simulink library "lib_all"  (Simulink 7.6)
settings: Solver=ode45  SolverMode=SingleTasking  StartTime=0.0  StopTime=10.0  RelTol=1e-3
values from neighbouring files (symbols this model uses):
  map_lib_03 = [6.8679 7.0159 7.4571 8.1849 9.1892 10.456 11.97 13.71 15.655 17.781 20.063 22.474 ... (803 elements, 73x11)]    [scr_simdata.mat]    (also in scr_simdata_all.mat)
  map_lib_06 = [2.5784 2.6727 2.9588 3.4453 4.1444 5.068 6.226 7.6242 9.2634 11.139 13.24 15.552 ... (803 elements, 73x11)]    [scr_simdata.mat]    (also in scr_simdata_all.mat)
  map_lib_09 = [0.0025784 0.0026727 0.0029588 0.0034453 0.0041444 0.005068 0.006226 0.0076242 0.0092634 0.011139 0.01324 0.015552 ... (803 elements, 73x11)]    [scr_simdata.mat]    (also in scr_simdata_all.mat)
  map_lib_10 = [2.74 2.75 2.76 2.77 2.78 2.79 2.8 2.81 2.82 2.83 2.84 2.85 ... (81 elements, 1x81)]    [scr_simdata.mat]    (also in scr_simdata_all.mat)
  map_lib_11 = [1.1983 1.2027 1.207 1.2113 1.2156 1.2198 1.2241 1.2283 1.2326 1.2368 1.241 1.2451 ... (81 elements, 1x81)]    [scr_simdata.mat]    (also in scr_simdata_all.mat)
  map_lib_12 = [2.74 2.75 2.76 2.77 2.78 2.79 2.8 2.81 2.82 2.83 2.84 2.85 ... (81 elements, 1x81)]    [scr_simdata.mat]    (also in scr_simdata_all.mat)
  map_lib_13 = [1.74 1.75 1.76 1.77 1.78 1.79 1.8 1.81 1.82 1.83 1.84 1.85 ... (81 elements, 1x81)]    [scr_simdata.mat]    (also in scr_simdata_all.mat)
  map_lib_16 = [0.00048828125 1.6159 1.8962 2.0821 2.2251 2.3426 2.4433 2.5318 2.611 2.683 2.749 2.8101 ... (1419 elements, 129x11)]    [scr_simdata.mat]    (also in scr_simdata_all.mat)
  map_mr2_01 = [0 800 1600 2400 3200 4000]    [scr_simdata.mat]    (also in scr_simdata_all.mat)
  map_mr2_02 = [1.32 1.32 1.33 1.34 1.36 1.38]    [scr_simdata.mat]    (also in scr_simdata_all.mat)
  map_mr2_03 = [0 800 1600 2400 3200 4000]    [scr_simdata.mat]    (also in scr_simdata_all.mat)
  map_mr2_04 = [1.32 1.32 1.33 1.34 1.36 1.38]    [scr_simdata.mat]    (also in scr_simdata_all.mat)
  map_mr2_05 = [0 800 1600 2400 3200 4000]    [scr_simdata.mat]    (also in scr_simdata_all.mat)
  map_mr2_06 = [1.32 1.32 1.33 1.34 1.36 1.38]    [scr_simdata.mat]    (also in scr_simdata_all.mat)
  Parameter1 = 6    [scr_simdata.mat]    (also in scr_simdata_all.mat)
  Parameter101 = 30    [scr_simdata.mat]    (also in scr_simdata_all.mat)
  Parameter102 = 12    [scr_simdata.mat]    (also in scr_simdata_all.mat)
  Parameter103 = -240    [scr_simdata.mat]    (also in scr_simdata_all.mat)
  Parameter108 = 48    [scr_simdata.mat]    (also in scr_simdata_all.mat)
  Parameter120 = 16    [scr_simdata.mat]    (also in scr_simdata_all.mat)
  Parameter121 = 87    [scr_simdata.mat]    (also in scr_simdata_all.mat)
  Parameter123 = 78    [scr_simdata.mat]    (also in scr_simdata_all.mat)
  Parameter124 = 60    [scr_simdata.mat]    (also in scr_simdata_all.mat)
  Parameter125 = -480    [scr_simdata.mat]    (also in scr_simdata_all.mat)
  Parameter130 = 96    [scr_simdata.mat]    (also in scr_simdata_all.mat)
  Parameter131 = 3    [scr_simdata.mat]    (also in scr_simdata_all.mat)
  Parameter144 = 3    [scr_simdata.mat]    (also in scr_simdata_all.mat)
  Parameter18 = 128    [scr_simdata.mat]    (also in scr_simdata_all.mat)
  Parameter19 = 16    [scr_simdata.mat]    (also in scr_simdata_all.mat)
  Parameter2 = 12    [scr_simdata.mat]    (also in scr_simdata_all.mat)
  Parameter20 = 7    [scr_simdata.mat]    (also in scr_simdata_all.mat)
  Parameter21 = 4    [scr_simdata.mat]    (also in scr_simdata_all.mat)
  Parameter3 = 18    [scr_simdata.mat]    (also in scr_simdata_all.mat)
  Parameter32 = 1    [scr_simdata.mat]    (also in scr_simdata_all.mat)
  Parameter34 = 24    [scr_simdata.mat]    (also in scr_simdata_all.mat)
  Parameter4 = 138    [scr_simdata.mat]    (also in scr_simdata_all.mat)
  Parameter42 = 48    [scr_simdata.mat]    (also in scr_simdata_all.mat)
  Parameter5 = 135    [scr_simdata.mat]    (also in scr_simdata_all.mat)
  Parameter55 = 0    [scr_simdata.mat]    (also in scr_simdata_all.mat)
  Parameter56 = 0    [scr_simdata.mat]    (also in scr_simdata_all.mat)
  Parameter57 = 1    [scr_simdata.mat]    (also in scr_simdata_all.mat)
  Parameter58 = 1    [scr_simdata.mat]    (also in scr_simdata_all.mat)
  Parameter59 = 2    [scr_simdata.mat]    (also in scr_simdata_all.mat)
  Parameter6 = 132    [scr_simdata.mat]    (also in scr_simdata_all.mat)
  Parameter60 = 2    [scr_simdata.mat]    (also in scr_simdata_all.mat)
  Parameter63 = 144    [scr_simdata.mat]    (also in scr_simdata_all.mat)
  Parameter7 = 126    [scr_simdata.mat]    (also in scr_simdata_all.mat)
  Parameter76 = 64    [scr_simdata.mat]    (also in scr_simdata_all.mat)
  Parameter77 = 135    [scr_simdata.mat]    (also in scr_simdata_all.mat)
  Parameter79 = 126    [scr_simdata.mat]    (also in scr_simdata_all.mat)
  Parameter80 = 108    [scr_simdata.mat]    (also in scr_simdata_all.mat)
  Parameter81 = 0    [scr_simdata.mat]    (also in scr_simdata_all.mat)
  Parameter86 = 0    [scr_simdata.mat]    (also in scr_simdata_all.mat)
  Parameter98 = 112    [scr_simdata.mat]    (also in scr_simdata_all.mat)
  Parameter99 = 39    [scr_simdata.mat]    (also in scr_simdata_all.mat)
undefined symbols (not defined in the file or in its folder): cylvolkp_hih_map2h, cylvolkp_map2h, pk1k_map2h
libraries: simulink x2
(part 2 of 36)

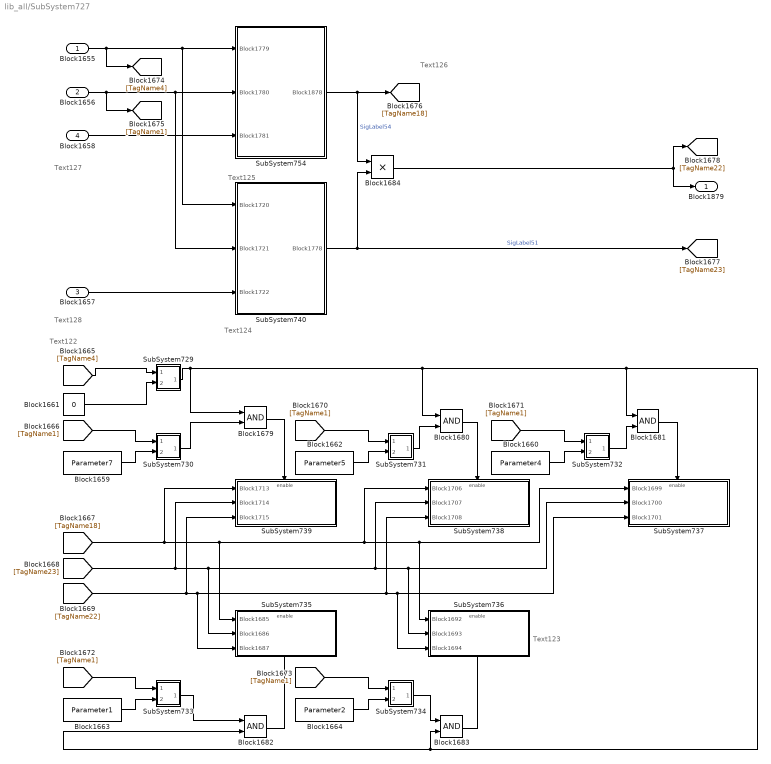
== system lib_all/SubSystem727 ==
  note: Text128
  note: Text127
  note: Text126
  note: Text125
  note: Text124
  note: Text123
  note: Text122
  Block1655 : Inport
  Block1656 : Inport  Port=2
  Block1657 : Inport  Port=3
  Block1658 : Inport  Port=4
  Block1659 : Constant  Value=Parameter7  OutDataTypeStr=double  SampleTime=-1
  Block1660 : Constant  Value=Parameter4  OutDataTypeStr=double  SampleTime=-1
  Block1661 : Constant  Value=0  OutDataTypeStr=double  SampleTime=-1
  Block1662 : Constant  Value=Parameter5  OutDataTypeStr=double  SampleTime=-1
  Block1663 : Constant  Value=Parameter1  OutDataTypeStr=double  SampleTime=-1
  Block1664 : Constant  Value=Parameter2  OutDataTypeStr=double  SampleTime=-1
  Block{1679..1683} (5 alike) : Logic
  Block1684 : Product  OutDataTypeStr=double  SaturateOnIntegerOverflow=on  RndMeth=Zero  InputSameDT=on
  SubSystem729 : Subsystem
  SubSystem730 : Subsystem
  SubSystem731 : Subsystem
  SubSystem732 : Subsystem
  SubSystem733 : Subsystem
  SubSystem734 : Subsystem
  SubSystem735 : Subsystem
      settings: PropExecContextOutsideSubsystem=on
  SubSystem736 : Subsystem
      settings: PropExecContextOutsideSubsystem=on
  SubSystem737 : Subsystem
      settings: PropExecContextOutsideSubsystem=on
  SubSystem738 : Subsystem
      settings: PropExecContextOutsideSubsystem=on
  SubSystem739 : Subsystem
      settings: PropExecContextOutsideSubsystem=on
  SubSystem740 : Subsystem  Priority=2  TreatAsAtomicUnit=on
      settings: AttributesFormatString=Attribute349  RTWFcnNameOpts=Use subsystem name
  SubSystem754 : Subsystem  Priority=1  TreatAsAtomicUnit=on
      settings: AttributesFormatString=Attribute363  RTWFcnNameOpts=Use subsystem name
  Block1879 : Outport
  wires:
  Block1657/1 -> SubSystem740/3(Block1722)
  Block1658/1 -> SubSystem754/3(Block1781)
  Block1655/1 -> [TagName4], SubSystem754/1(Block1779), SubSystem740/1(Block1720)
  Block1656/1 -> [TagName1], SubSystem754/2(Block1780), SubSystem740/2(Block1721)
  SubSystem754/1(Block1878) -> [TagName18], Block1684/1  "SigLabel54"
  SubSystem740/1(Block1778) -> [TagName23], Block1684/2  "SigLabel51"
  Block1684/1 -> [TagName22], Block1879/1
  [TagName4] -> SubSystem729/1(In1)
  Block1661/1 -> SubSystem729/2(In2)
  [TagName1] -> SubSystem730/1(In1)
  Block1659/1 -> SubSystem730/2(In2)
  SubSystem729/1(Out1) -> Block1682/2, Block1683/2, Block1681/1, Block1680/1, Block1679/1
  SubSystem730/1(Out1) -> Block1679/2
  [TagName1] -> SubSystem731/1(In1)
  Block1662/1 -> SubSystem731/2(In2)
  SubSystem731/1(Out1) -> Block1680/2
  Block1679/1 -> SubSystem739/Enable
  [TagName1] -> SubSystem732/1(In1)
  Block1660/1 -> SubSystem732/2(In2)
  SubSystem732/1(Out1) -> Block1681/2
  Block1680/1 -> SubSystem738/Enable
  Block1681/1 -> SubSystem737/Enable
  [TagName18] -> SubSystem738/1(Block1706), SubSystem736/1(Block1692), SubSystem737/1(Block1699), SubSystem735/1(Block1685), SubSystem739/1(Block1713)
  [TagName23] -> SubSystem738/2(Block1707), SubSystem736/2(Block1693), SubSystem737/2(Block1700), SubSystem735/2(Block1686), SubSystem739/2(Block1714)
  [TagName22] -> SubSystem738/3(Block1708), SubSystem736/3(Block1694), SubSystem737/3(Block1701), SubSystem735/3(Block1687), SubSystem739/3(Block1715)
  [TagName1] -> SubSystem733/1(In1)
  Block1663/1 -> SubSystem733/2(In2)
  [TagName1] -> SubSystem734/1(In1)
  Block1664/1 -> SubSystem734/2(In2)
  Block1682/1 -> SubSystem735/Enable
  Block1683/1 -> SubSystem736/Enable
  SubSystem733/1(Out1) -> Block1682/1
  SubSystem734/1(Out1) -> Block1683/1

== system lib_all/SubSystem795 ==
  note: Text129
  Block1880 : Inport
  Block1881 : Inport  Port=2
  Block1882 : Inport  Port=3
  Block{1883,1885,1887..1888} (4 alike) : Constant  OutDataTypeStr=double  SampleTime=-1
  Block1884 : Constant  Value=0  OutDataTypeStr=double  SampleTime=-1
  Block1886 : Constant  Value=0  OutDataTypeStr=double  SampleTime=-1
  Block1894 : Sum  OutDataTypeStr=double  RndMeth=Zero  SaturateOnIntegerOverflow=on  InputSameDT=on
  Block1895 : Sum  Inputs=+++  OutDataTypeStr=double  RndMeth=Zero  SaturateOnIntegerOverflow=on  InputSameDT=on
  Block1896 : Sum  Inputs=+++  OutDataTypeStr=double  RndMeth=Zero  SaturateOnIntegerOverflow=on  InputSameDT=on
  Block1897 : Sum  Inputs=--+  OutDataTypeStr=double  RndMeth=Zero  SaturateOnIntegerOverflow=on  InputSameDT=on
  Block1898 : Sum  Inputs=--+  OutDataTypeStr=double  RndMeth=Zero  SaturateOnIntegerOverflow=on  InputSameDT=on
  Block{1899..1902} (4 alike) : Switch  Threshold=0.5  RndMeth=Zero  SaturateOnIntegerOverflow=on  InputSameDT=on
  SubSystem796 : Subsystem
  SubSystem797 : Subsystem
  SubSystem798 : Subsystem
  SubSystem799 : Subsystem
  Block1903 : Outport
  wires:
  Block1880/1 -> [TagName14]
  SubSystem796/1(Out1) -> Block1900/2
  Block1884/1 -> SubSystem796/1(In1)
  Block1895/1 -> Block1896/3, Block1899/3, SubSystem797/2(In2)
  Block1883/1 -> Block1895/2
  [TagName14] -> Block1895/1, Block1896/1
  SubSystem797/1(Out1) -> Block1899/2
  Block1886/1 -> SubSystem797/1(In1)
  Block1896/1 -> Block1899/1
  Block1885/1 -> Block1896/2
  Block1899/1 -> Block1900/1
  SubSystem798/1(Out1) -> Block1901/2
  [TagName14] -> SubSystem798/1(In1)
  Block1897/1 -> Block1901/1
  Block1887/1 -> Block1897/2
  [TagName14] -> Block1897/1, Block1898/1
  SubSystem799/1(Out1) -> Block1902/2
  [TagName14] -> SubSystem799/1(In1)
  Block1901/1 -> Block1898/3, Block1902/3, SubSystem799/2(In2)
  Block1898/1 -> Block1902/1
  Block1888/1 -> Block1898/2
  Block1902/1 -> Block1900/3
  Block1881/1 -> Block1894/1
  Block1882/1 -> Block1894/2
  Block1900/1 -> Block1903/1
  Block1894/1 -> Block1895/3, SubSystem796/2(In2), Block1901/3, SubSystem798/2(In2), Block1897/3

== system lib_all/SubSystem800 ==
  note: Text178
  note: Text177
  note: Text176
  Block1904 : Inport
  Block1905 : Inport  Port=2
  Block1906 : Constant  OutDataTypeStr=double  SampleTime=-1
  Block1907 : DataStoreRead  DataStoreName=DSM191
  Block1908 : DataStoreRead  DataStoreName=DSM230
  Block1914 : Sum  Inputs=-+  OutDataTypeStr=double  RndMeth=Zero  SaturateOnIntegerOverflow=on  InputSameDT=on
  Block1915 : Sum  Inputs=++|  OutDataTypeStr=double  RndMeth=Zero  SaturateOnIntegerOverflow=on  InputSameDT=on
  SubSystem801 : Subsystem  TreatAsAtomicUnit=on  mask(***ATC.DSRVEC***){bd_version=1.01.A, r0=DSM182, r1=-1, r2=, r3=, r4=, r5=, r6=, r7=, r8=, r9=, c0=, c1=, c2=, c3=, c4=, c5=, c6=, c7=, c8=, c9=}
      mask prompts: bd_version "Version"
      mask help: これはAutoCからのブロックです．編集禁止．
      settings: OpenFcn=warndlg('変更禁止!');
      mask Help: web(ui_blockhelpmng(gcb));
      mask Display: disp('ATC.DSRVEC')
  SubSystem802 : Subsystem
  SubSystem803 : Subsystem  TreatAsAtomicUnit=on
  SubSystem819 : Subsystem  TreatAsAtomicUnit=on
      settings: AttributesFormatString=Attribute417  RTWFcnNameOpts=Use subsystem name
  SubSystem887 : Subsystem  TreatAsAtomicUnit=on
      settings: AttributesFormatString=Attribute454  RTWFcnNameOpts=Use subsystem name
  SubSystem955 : Subsystem  TreatAsAtomicUnit=on
  wires:
  Block1905/1 -> SubSystem803/2(Block1917), SubSystem887/2(Block2229)
  SubSystem803/1(Block2001) -> SubSystem819/2(Block2004)
  Block1908/1 -> SubSystem819/3(Block2005), SubSystem887/3(Block2230)
  Block1907/1 -> SubSystem819/4(Block2006), SubSystem887/4(Block2231)
  SubSystem819/1(Block2227) -> Block1914/1  "SigLabel82"
  SubSystem887/1(Block2452) -> Block1914/2, SubSystem955/3(Block2455)  "SigLabel81"
  SubSystem803/2(Block2002) -> [TagName12]
  Block1914/1 -> SubSystem802/2(In2), SubSystem955/2(Block2454)  "SigLabel78"
  [TagName13] -> SubSystem801/1(element)
  SubSystem801/1(Output) -> SubSystem802/1(In1)
  SubSystem802/1(Out1) -> SubSystem955/Enable
  [TagName13] -> SubSystem955/1(Block2453)
  [TagName12] -> SubSystem955/4(Block2456)
  Block1904/1 -> SubSystem819/1(Block2003), SubSystem887/1(Block2228), Block1915/1
  Block1915/1 -> SubSystem803/1(Block1916), [TagName13]
  Block1906/1 -> Block1915/2

== system lib_all/SubSystem959 ==
  note: Text188
  note: Text187
  Block2458 : Inport
  Block2459 : Inport  Port=2
  Block2460 : Constant  Value=3  OutDataTypeStr=double  SampleTime=-1
  Block2461 : Constant  Value=3  OutDataTypeStr=double  SampleTime=-1
  Block2462 : Constant  Value=3  OutDataTypeStr=double  SampleTime=-1
  Block2463 : Product  Inputs=*/  OutDataTypeStr=double  SaturateOnIntegerOverflow=on  RndMeth=Zero  InputSameDT=on
  Block2464 : Product  Inputs=*/  OutDataTypeStr=double  SaturateOnIntegerOverflow=on  RndMeth=Zero  InputSameDT=on
  Block2468 : Sum  Inputs=+-  OutDataTypeStr=double  RndMeth=Zero  SaturateOnIntegerOverflow=on  InputSameDT=on
  SubSystem961 : Subsystem  TreatAsAtomicUnit=on
      settings: RTWSystemCode=Inline
  SubSystem963 : Subsystem
  Block2622 : Outport
  wires:
  Block2458/1 -> SubSystem963/3(Block2471)
  Block2459/1 -> SubSystem963/2(Block2470)
  Block2468/1 -> Block2622/1
  Block2464/1 -> Block2468/2
  Block2463/1 -> Block2468/1
  Block2461/1 -> Block2464/2
  SubSystem963/2(Block2621) -> Block2464/1
  Block2460/1 -> Block2463/2
  SubSystem963/1(Block2620) -> Block2463/1
  SubSystem961/1(action) -> SubSystem963/Trigger
  SubSystem961/2(i) -> SubSystem963/1(Block2469)
  Block2462/1 -> SubSystem961/1(N)

== system lib_all/SubSystem1/SubSystem2 ==
  note: ***編集禁止***
  In1 : Inport
  In2 : Inport  Port=2
  LO1 : Logic  Operator=OR
  RO1 : RelationalOperator  InputSameDT=on
  RO2 : RelationalOperator  Operator===  InputSameDT=on
  single1 : DataTypeConversion  OutDataTypeStr=single  SaturateOnIntegerOverflow=on  RndMeth=Zero
  single2 : DataTypeConversion  OutDataTypeStr=single  SaturateOnIntegerOverflow=on  RndMeth=Zero
  Out1 : Outport
  wires:
  In2/1 -> single2/1
  In1/1 -> single1/1
  single1/1 -> RO1/1, RO2/1
  single2/1 -> RO1/2, RO2/2
  RO1/1 -> LO1/1
  RO2/1 -> LO1/2
  LO1/1 -> Out1/1

== system lib_all/SubSystem1023/SubSystem1024 ==
  note: ***編集禁止***
  mult : Inport
  div : Inport  Port=2
  Product : Product  Inputs=*/  SaturateOnIntegerOverflow=on  RndMeth=Zero  InputSameDT=on  OutDataTypeStr=Inherit: Same as first input
  Out : Outport
  wires:
  div/1 -> Product/2
  mult/1 -> Product/1
  Product/1 -> Out/1

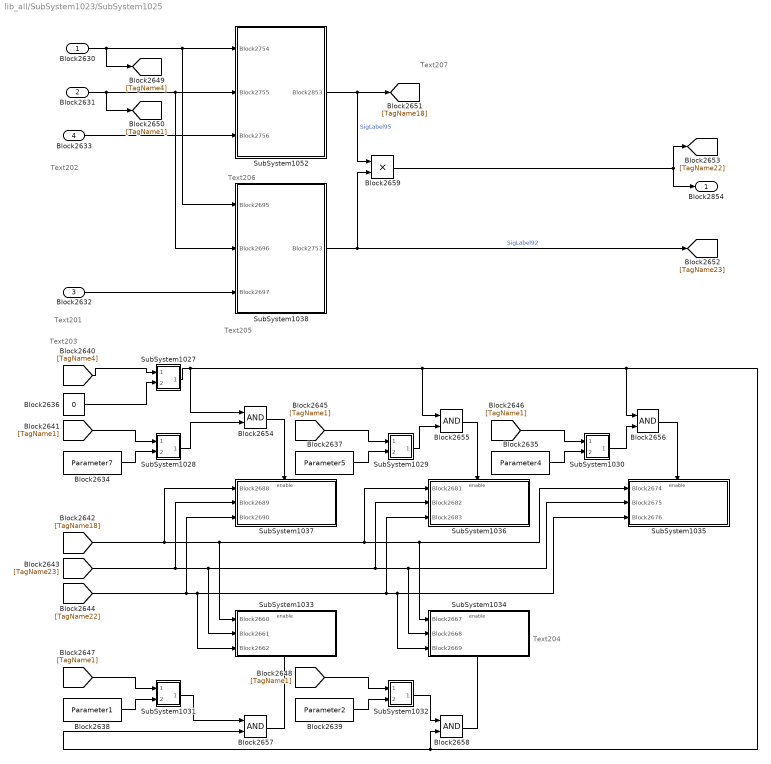
== system lib_all/SubSystem1023/SubSystem1025 ==
  note: Text207
  note: Text206
  note: Text205
  note: Text204
  note: Text203
  note: Text202
  note: Text201
  Block2630 : Inport
  Block2631 : Inport  Port=2
  Block2632 : Inport  Port=3
  Block2633 : Inport  Port=4
  Block2634 : Constant  Value=Parameter7  OutDataTypeStr=double  SampleTime=-1
  Block2635 : Constant  Value=Parameter4  OutDataTypeStr=double  SampleTime=-1
  Block2636 : Constant  Value=0  OutDataTypeStr=double  SampleTime=-1
  Block2637 : Constant  Value=Parameter5  OutDataTypeStr=double  SampleTime=-1
  Block2638 : Constant  Value=Parameter1  OutDataTypeStr=double  SampleTime=-1
  Block2639 : Constant  Value=Parameter2  OutDataTypeStr=double  SampleTime=-1
  Block{2654..2658} (5 alike) : Logic
  Block2659 : Product  OutDataTypeStr=double  SaturateOnIntegerOverflow=on  RndMeth=Zero  InputSameDT=on
  SubSystem1027 : Subsystem
  SubSystem1028 : Subsystem
  SubSystem1029 : Subsystem
  SubSystem1030 : Subsystem
  SubSystem1031 : Subsystem
  SubSystem1032 : Subsystem
  SubSystem1033 : Subsystem
      settings: PropExecContextOutsideSubsystem=on
  SubSystem1034 : Subsystem
      settings: PropExecContextOutsideSubsystem=on
  SubSystem1035 : Subsystem
      settings: PropExecContextOutsideSubsystem=on
  SubSystem1036 : Subsystem
      settings: PropExecContextOutsideSubsystem=on
  SubSystem1037 : Subsystem
      settings: PropExecContextOutsideSubsystem=on
  SubSystem1038 : Subsystem  Priority=2  TreatAsAtomicUnit=on
      settings: AttributesFormatString=Attribute533  RTWFcnNameOpts=Use subsystem name
  SubSystem1052 : Subsystem  Priority=1  TreatAsAtomicUnit=on
      settings: AttributesFormatString=Attribute547  RTWFcnNameOpts=Use subsystem name
  Block2854 : Outport
  wires:
  Block2630/1 -> [TagName4], SubSystem1052/1(Block2754), SubSystem1038/1(Block2695)
  Block2631/1 -> [TagName1], SubSystem1052/2(Block2755), SubSystem1038/2(Block2696)
  SubSystem1052/1(Block2853) -> [TagName18], Block2659/1  "SigLabel95"
  SubSystem1038/1(Block2753) -> [TagName23], Block2659/2  "SigLabel92"
  Block2659/1 -> [TagName22], Block2854/1
  [TagName4] -> SubSystem1027/1(In1)
  Block2636/1 -> SubSystem1027/2(In2)
  [TagName1] -> SubSystem1028/1(In1)
  Block2634/1 -> SubSystem1028/2(In2)
  SubSystem1027/1(Out1) -> Block2657/2, Block2658/2, Block2656/1, Block2655/1, Block2654/1
  SubSystem1028/1(Out1) -> Block2654/2
  [TagName1] -> SubSystem1029/1(In1)
  Block2637/1 -> SubSystem1029/2(In2)
  SubSystem1029/1(Out1) -> Block2655/2
  Block2654/1 -> SubSystem1037/Enable
  [TagName1] -> SubSystem1030/1(In1)
  Block2635/1 -> SubSystem1030/2(In2)
  SubSystem1030/1(Out1) -> Block2656/2
  Block2655/1 -> SubSystem1036/Enable
  Block2656/1 -> SubSystem1035/Enable
  [TagName18] -> SubSystem1036/1(Block2681), SubSystem1034/1(Block2667), SubSystem1035/1(Block2674), SubSystem1033/1(Block2660), SubSystem1037/1(Block2688)
  [TagName23] -> SubSystem1036/2(Block2682), SubSystem1034/2(Block2668), SubSystem1035/2(Block2675), SubSystem1033/2(Block2661), SubSystem1037/2(Block2689)
  [TagName22] -> SubSystem1036/3(Block2683), SubSystem1034/3(Block2669), SubSystem1035/3(Block2676), SubSystem1033/3(Block2662), SubSystem1037/3(Block2690)
  [TagName1] -> SubSystem1031/1(In1)
  Block2638/1 -> SubSystem1031/2(In2)
  [TagName1] -> SubSystem1032/1(In1)
  Block2639/1 -> SubSystem1032/2(In2)
  Block2657/1 -> SubSystem1033/Enable
  Block2658/1 -> SubSystem1034/Enable
  SubSystem1031/1(Out1) -> Block2657/1
  SubSystem1032/1(Out1) -> Block2658/1
  Block2632/1 -> SubSystem1038/3(Block2697)
  Block2633/1 -> SubSystem1052/3(Block2756)

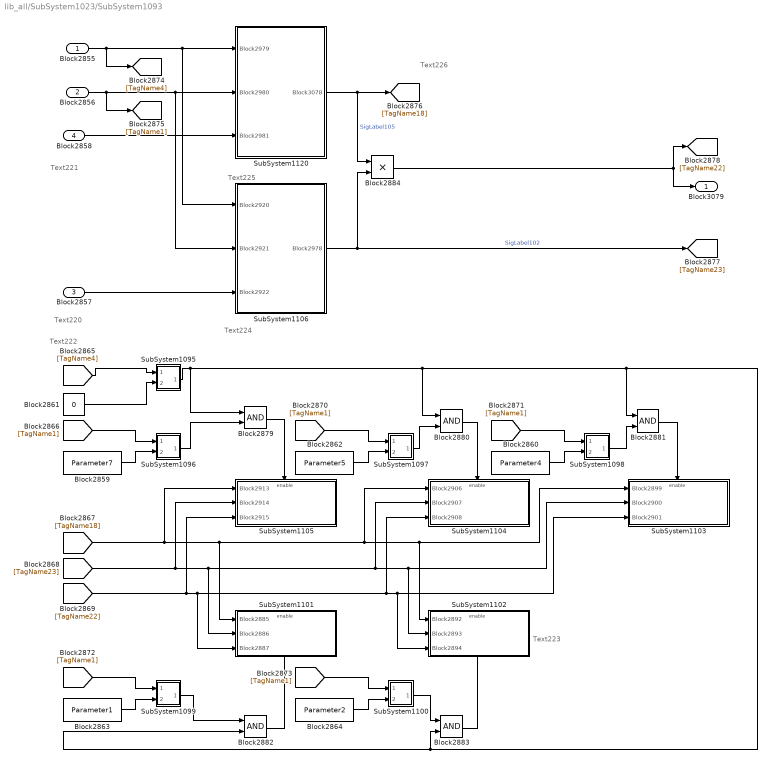
== system lib_all/SubSystem1023/SubSystem1093 ==
  note: Text226
  note: Text225
  note: Text224
  note: Text223
  note: Text222
  note: Text221
  note: Text220
  Block2855 : Inport
  Block2856 : Inport  Port=2
  Block2857 : Inport  Port=3
  Block2858 : Inport  Port=4
  Block2859 : Constant  Value=Parameter7  OutDataTypeStr=double  SampleTime=-1
  Block2860 : Constant  Value=Parameter4  OutDataTypeStr=double  SampleTime=-1
  Block2861 : Constant  Value=0  OutDataTypeStr=double  SampleTime=-1
  Block2862 : Constant  Value=Parameter5  OutDataTypeStr=double  SampleTime=-1
  Block2863 : Constant  Value=Parameter1  OutDataTypeStr=double  SampleTime=-1
  Block2864 : Constant  Value=Parameter2  OutDataTypeStr=double  SampleTime=-1
  Block{2879..2883} (5 alike) : Logic
  Block2884 : Product  OutDataTypeStr=double  SaturateOnIntegerOverflow=on  RndMeth=Zero  InputSameDT=on
  SubSystem1095 : Subsystem
  SubSystem1096 : Subsystem
  SubSystem1097 : Subsystem
  SubSystem1098 : Subsystem
  SubSystem1099 : Subsystem
  SubSystem1100 : Subsystem
  SubSystem1101 : Subsystem
      settings: PropExecContextOutsideSubsystem=on
  SubSystem1102 : Subsystem
      settings: PropExecContextOutsideSubsystem=on
  SubSystem1103 : Subsystem
      settings: PropExecContextOutsideSubsystem=on
  SubSystem1104 : Subsystem
      settings: PropExecContextOutsideSubsystem=on
  SubSystem1105 : Subsystem
      settings: PropExecContextOutsideSubsystem=on
  SubSystem1106 : Subsystem  Priority=2  TreatAsAtomicUnit=on
      settings: AttributesFormatString=Attribute570  RTWFcnNameOpts=Use subsystem name
  SubSystem1120 : Subsystem  Priority=1  TreatAsAtomicUnit=on
      settings: AttributesFormatString=Attribute584  RTWFcnNameOpts=Use subsystem name
  Block3079 : Outport
  wires:
  Block2855/1 -> [TagName4], SubSystem1120/1(Block2979), SubSystem1106/1(Block2920)
  Block2856/1 -> [TagName1], SubSystem1120/2(Block2980), SubSystem1106/2(Block2921)
  SubSystem1120/1(Block3078) -> [TagName18], Block2884/1  "SigLabel105"
  SubSystem1106/1(Block2978) -> [TagName23], Block2884/2  "SigLabel102"
  Block2884/1 -> [TagName22], Block3079/1
  [TagName4] -> SubSystem1095/1(In1)
  Block2861/1 -> SubSystem1095/2(In2)
  [TagName1] -> SubSystem1096/1(In1)
  Block2859/1 -> SubSystem1096/2(In2)
  SubSystem1095/1(Out1) -> Block2882/2, Block2883/2, Block2881/1, Block2880/1, Block2879/1
  SubSystem1096/1(Out1) -> Block2879/2
  [TagName1] -> SubSystem1097/1(In1)
  Block2862/1 -> SubSystem1097/2(In2)
  SubSystem1097/1(Out1) -> Block2880/2
  Block2879/1 -> SubSystem1105/Enable
  [TagName1] -> SubSystem1098/1(In1)
  Block2860/1 -> SubSystem1098/2(In2)
  SubSystem1098/1(Out1) -> Block2881/2
  Block2880/1 -> SubSystem1104/Enable
  Block2881/1 -> SubSystem1103/Enable
  [TagName18] -> SubSystem1104/1(Block2906), SubSystem1102/1(Block2892), SubSystem1103/1(Block2899), SubSystem1101/1(Block2885), SubSystem1105/1(Block2913)
  [TagName23] -> SubSystem1104/2(Block2907), SubSystem1102/2(Block2893), SubSystem1103/2(Block2900), SubSystem1101/2(Block2886), SubSystem1105/2(Block2914)
  [TagName22] -> SubSystem1104/3(Block2908), SubSystem1102/3(Block2894), SubSystem1103/3(Block2901), SubSystem1101/3(Block2887), SubSystem1105/3(Block2915)
  [TagName1] -> SubSystem1099/1(In1)
  Block2863/1 -> SubSystem1099/2(In2)
  [TagName1] -> SubSystem1100/1(In1)
  Block2864/1 -> SubSystem1100/2(In2)
  Block2882/1 -> SubSystem1101/Enable
  Block2883/1 -> SubSystem1102/Enable
  SubSystem1099/1(Out1) -> Block2882/1
  SubSystem1100/1(Out1) -> Block2883/1
  Block2857/1 -> SubSystem1106/3(Block2922)
  Block2858/1 -> SubSystem1120/3(Block2981)

== system lib_all/SubSystem1023/SubSystem1161 ==
  note: Text230
  note: Text229
  Block3080 : Inport
  Block3081 : Inport  Port=2
  Block3082 : Inport  Port=3
  Block3083 : Constant  Value=0  OutDataTypeStr=double  SampleTime=-1
  SubSystem1163 : Subsystem
  SubSystem1164 : Subsystem
  SubSystem1172 : Subsystem  TreatAsAtomicUnit=on
  SubSystem1173 : Subsystem
  Block3138 : Outport
  wires:
  [TagName23] -> SubSystem1172/3(Block3125)
  [TagName11] -> SubSystem1172/2(Block3124)
  [TagName1] -> SubSystem1172/1(Block3123)
  SubSystem1163/1(Out1) -> SubSystem1172/Enable
  Block3083/1 -> SubSystem1163/2(In2)
  [TagName4] -> SubSystem1163/1(In1)
  SubSystem1173/1(Block3137) -> Block3138/1, [TagName23]
  Block3082/1 -> SubSystem1173/1(Block3130)
  SubSystem1164/1(Block3122) -> SubSystem1173/2(Block3131), [TagName11]
  Block3080/1 -> SubSystem1164/2(Block3093), [TagName4]
  Block3081/1 -> SubSystem1164/1(Block3092), [TagName1]

== system lib_all/SubSystem1023/SubSystem1175 ==
  note: Text234
  note: Text233
  Block3139 : Inport
  Block3140 : Inport  Port=2
  Block3141 : Inport  Port=3
  Block3142 : Constant  Value=0  OutDataTypeStr=double  SampleTime=-1
  SubSystem1177 : Subsystem
  SubSystem1178 : Subsystem
  SubSystem1186 : Subsystem  TreatAsAtomicUnit=on
  SubSystem1187 : Subsystem
  Block3197 : Outport
  wires:
  [TagName23] -> SubSystem1186/3(Block3184)
  [TagName11] -> SubSystem1186/2(Block3183)
  [TagName1] -> SubSystem1186/1(Block3182)
  SubSystem1177/1(Out1) -> SubSystem1186/Enable
  Block3142/1 -> SubSystem1177/2(In2)
  [TagName4] -> SubSystem1177/1(In1)
  SubSystem1187/1(Block3196) -> Block3197/1, [TagName23]
  Block3141/1 -> SubSystem1187/1(Block3189)
  SubSystem1178/1(Block3181) -> SubSystem1187/2(Block3190), [TagName11]
  Block3139/1 -> SubSystem1178/2(Block3152), [TagName4]
  Block3140/1 -> SubSystem1178/1(Block3151), [TagName1]

== system lib_all/SubSystem1023/SubSystem1189 ==
  note: Text235
  Block3198 : Inport
  Block3199 : Constant  Value=0  OutDataTypeStr=double  SampleTime=-1
  Block3200 : Constant  Value=0  OutDataTypeStr=double  SampleTime=-1
  Block3201 : Constant  OutDataTypeStr=double  SampleTime=-1
  Block3202 : Constant  OutDataTypeStr=double  SampleTime=-1
  Block3203 : Constant  OutDataTypeStr=double  SampleTime=-1
  Block3204 : DataStoreRead  DataStoreName=DSM48
  Block3205 : DataStoreRead  DataStoreName=DSM38
  Block3206 : DataStoreRead  DataStoreName=DSM37
  Block3207 : Sum  OutDataTypeStr=double  RndMeth=Zero  SaturateOnIntegerOverflow=on  InputSameDT=on
  Block{3208..3211} (4 alike) : Switch  Threshold=0.5  RndMeth=Zero  SaturateOnIntegerOverflow=on  InputSameDT=on
  Block3212 : Lookup  InputValues=map_mr2_01  Table=map_mr2_02  SaturateOnIntegerOverflow=on
      settings: AttributesFormatString=<%<OutputValues>>\nAttribute633
  Block3213 : Lookup  InputValues=map_mr2_03  Table=map_mr2_04  SaturateOnIntegerOverflow=on
      settings: AttributesFormatString=<%<OutputValues>>\nAttribute634
  Block3214 : Lookup  InputValues=map_mr2_05  Table=map_mr2_06  SaturateOnIntegerOverflow=on
      settings: AttributesFormatString=<%<OutputValues>>\nAttribute635
  SubSystem1190 : Subsystem
  SubSystem1191 : Subsystem
  SubSystem1192 : Subsystem
  SubSystem1193 : Subsystem
  SubSystem1194 : Subsystem  TreatAsAtomicUnit=on  mask(***ATC.DSRVEC***){bd_version=1.01.A, r0=DSM97, r1=-1, r2=, r3=, r4=, r5=, r6=, r7=, r8=, r9=, c0=, c1=, c2=, c3=, c4=, c5=, c6=, c7=, c8=, c9=}
      mask prompts: bd_version "Version"
      mask help: これはAutoCからのブロックです．編集禁止．
      settings: OpenFcn=warndlg('変更禁止!');
      mask Help: web(ui_blockhelpmng(gcb));
      mask Display: disp('ATC.DSRVEC')
  SubSystem1195 : Subsystem  TreatAsAtomicUnit=on  mask(***ATC.DSRVEC***){bd_version=1.01.A, r0=DSM9, r1=-1, r2=, r3=, r4=, r5=, r6=, r7=, r8=, r9=, c0=, c1=, c2=, c3=, c4=, c5=, c6=, c7=, c8=, c9=}
      mask prompts: bd_version "Version"
      mask help: これはAutoCからのブロックです．編集禁止．
      settings: OpenFcn=warndlg('変更禁止!');
      mask Help: web(ui_blockhelpmng(gcb));
      mask Display: disp('ATC.DSRVEC')
  Block3215 : Outport
  wires:
  Block3207/1 -> SubSystem1195/1(element), SubSystem1194/1(element)
  Block3198/1 -> Block3207/2
  Block3203/1 -> Block3207/1
  SubSystem1194/1(Output) -> Block3213/1, Block3212/1, Block3214/1
  SubSystem1190/1(Out1) -> Block3208/2
  SubSystem1191/1(Out1) -> Block3209/2
  Block3213/1 -> Block3209/1
  Block3205/1 -> SubSystem1191/2(In2), SubSystem1192/1(In1)
  Block3200/1 -> SubSystem1191/1(In1)
  Block3201/1 -> SubSystem1192/2(In2)
  SubSystem1192/1(Out1) -> Block3210/2
  Block3212/1 -> Block3210/1
  Block3214/1 -> Block3210/3
  Block3199/1 -> SubSystem1190/1(In1)
  Block3204/1 -> SubSystem1190/2(In2), SubSystem1193/1(In1)
  Block3202/1 -> SubSystem1193/2(In2)
  SubSystem1193/1(Out1) -> Block3211/2
  Block3206/1 -> Block3211/1
  SubSystem1195/1(Output) -> Block3211/3
  Block3208/1 -> Block3215/1
  Block3209/1 -> Block3208/1
  Block3211/1 -> Block3208/3
  Block3210/1 -> Block3209/3

== system lib_all/SubSystem1196/SubSystem1198 ==
  note: ***編集禁止***
  In1 : Inport
  In2 : Inport  Port=2
  RO1 : RelationalOperator  Operator===  InputSameDT=on
  single1 : DataTypeConversion  OutDataTypeStr=single  SaturateOnIntegerOverflow=on  RndMeth=Zero
  single2 : DataTypeConversion  OutDataTypeStr=single  SaturateOnIntegerOverflow=on  RndMeth=Zero
  Out1 : Outport
  wires:
  RO1/1 -> Out1/1
  In2/1 -> single2/1
  In1/1 -> single1/1
  single1/1 -> RO1/1
  single2/1 -> RO1/2

== system lib_all/SubSystem1196/SubSystem1199 ==
  Block3241 : Inport
  Block3242 : Inport  Port=2
  Block3243 : Inport  Port=3
  Block3244 : Inport  Port=4
  Block3245 : Inport  Port=5
  Block3246 : Inport  Port=6
  Block3247 : EnablePort
  Block3248 : DataStoreWrite  DataStoreName=DSM85
  Block3249 : DataStoreWrite  DataStoreName=DSM52
  Block3250 : DataStoreWrite  DataStoreName=DSM88
  Block3251 : DataStoreWrite  DataStoreName=DSM93
  Block3252 : DataStoreWrite  DataStoreName=DSM94
  Block3253 : DataStoreWrite  DataStoreName=DSM96
  wires:
  Block3241/1 -> Block3248/1
  Block3242/1 -> Block3250/1
  Block3243/1 -> Block3251/1
  Block3244/1 -> Block3252/1
  Block3245/1 -> Block3253/1
  Block3246/1 -> Block3249/1

== system lib_all/SubSystem1196/SubSystem1200 ==
  Block3256 : Inport
  Block3257 : Constant  Value=0  OutDataTypeStr=double  SampleTime=-1
  Block3258 : Constant  Value=0  OutDataTypeStr=double  SampleTime=-1
  Block3259 : Constant  OutDataTypeStr=double  SampleTime=-1
  Block3260 : Constant  OutDataTypeStr=double  SampleTime=-1
  Block3261 : DataStoreRead  DataStoreName=DSM38
  Block3262 : DataStoreRead  DataStoreName=DSM37
  Block3263 : DataStoreRead  DataStoreName=DSM48
  Block3264 : Sum  OutDataTypeStr=double  RndMeth=Zero  SaturateOnIntegerOverflow=on  InputSameDT=on
  Block3265 : Switch  Threshold=0.5  RndMeth=Zero  SaturateOnIntegerOverflow=on  InputSameDT=on
  Block3266 : Switch  Threshold=0.5  RndMeth=Zero  SaturateOnIntegerOverflow=on  InputSameDT=on
  Block3267 : Switch  Threshold=0.5  RndMeth=Zero  SaturateOnIntegerOverflow=on  InputSameDT=on
  Block3268 : Lookup  InputValues=map_mr2_01  Table=map_mr2_02  SaturateOnIntegerOverflow=on
      settings: AttributesFormatString=<%<OutputValues>>\nAttribute645
  Block3269 : Lookup  InputValues=map_mr2_03  Table=map_mr2_04  SaturateOnIntegerOverflow=on
      settings: AttributesFormatString=<%<OutputValues>>\nAttribute646
  SubSystem1201 : Subsystem
  SubSystem1202 : Subsystem
  SubSystem1203 : Subsystem
  SubSystem1204 : Subsystem  TreatAsAtomicUnit=on  mask(***ATC.DSRVEC***){bd_version=1.01.A, r0=DSM97, r1=-1, r2=, r3=, r4=, r5=, r6=, r7=, r8=, r9=, c0=, c1=, c2=, c3=, c4=, c5=, c6=, c7=, c8=, c9=}
      mask prompts: bd_version "Version"
      mask help: これはAutoCからのブロックです．編集禁止．
      settings: OpenFcn=warndlg('変更禁止!');
      mask Help: web(ui_blockhelpmng(gcb));
      mask Display: disp('ATC.DSRVEC')
  SubSystem1205 : Subsystem  TreatAsAtomicUnit=on  mask(***ATC.DSRVEC***){bd_version=1.01.A, r0=DSM9, r1=-1, r2=, r3=, r4=, r5=, r6=, r7=, r8=, r9=, c0=, c1=, c2=, c3=, c4=, c5=, c6=, c7=, c8=, c9=}
      mask prompts: bd_version "Version"
      mask help: これはAutoCからのブロックです．編集禁止．
      settings: OpenFcn=warndlg('変更禁止!');
      mask Help: web(ui_blockhelpmng(gcb));
      mask Display: disp('ATC.DSRVEC')
  Block3270 : Outport
  wires:
  SubSystem1201/1(Out1) -> Block3265/2
  Block3261/1 -> SubSystem1202/1(In1)
  Block3258/1 -> SubSystem1202/2(In2)
  SubSystem1202/1(Out1) -> Block3266/2
  Block3264/1 -> SubSystem1205/1(element), SubSystem1204/1(element)
  SubSystem1204/1(Output) -> Block3268/1, Block3269/1
  Block3269/1 -> Block3266/1
  Block3268/1 -> Block3266/3
  Block3257/1 -> SubSystem1201/1(In1)
  Block3263/1 -> SubSystem1201/2(In2), SubSystem1203/1(In1)
  Block3259/1 -> SubSystem1203/2(In2)
  SubSystem1203/1(Out1) -> Block3267/2
  Block3266/1 -> Block3265/1
  Block3267/1 -> Block3265/3
  Block3262/1 -> Block3267/1
  SubSystem1205/1(Output) -> Block3267/3
  Block3256/1 -> Block3264/1
  Block3265/1 -> Block3270/1
  Block3260/1 -> Block3264/2

== system lib_all/SubSystem1196/SubSystem1206 ==
  note: Text248
  note: Text247
  note: Text246
  Block3271 : Inport
  Block3272 : Inport  Port=2
  Block3273 : Inport  Port=3
  Block3274 : Inport  Port=4
  Block3275 : Inport  Port=5
  Block3276 : Product  OutDataTypeStr=double  SaturateOnIntegerOverflow=on  RndMeth=Zero  InputSameDT=on
  SubSystem1208 : Subsystem  Priority=1  TreatAsAtomicUnit=on
      settings: AttributesFormatString=Attribute648  RTWFcnNameOpts=Use subsystem name
  SubSystem1261 : Subsystem  Priority=2  TreatAsAtomicUnit=on
      settings: AttributesFormatString=Attribute667  RTWFcnNameOpts=Use subsystem name
  Block3445 : Outport
  wires:
  Block3271/1 -> SubSystem1261/1(Block3399), SubSystem1208/1(Block3277)
  Block3272/1 -> SubSystem1261/2(Block3400), SubSystem1208/2(Block3278)
  SubSystem1208/1(Block3398) -> Block3276/1  "SigLabel113"
  SubSystem1261/1(Block3444) -> Block3276/2  "SigLabel112"
  Block3276/1 -> Block3445/1
  Block3273/1 -> SubSystem1261/3(Block3401), SubSystem1208/3(Block3279)
  Block3275/1 -> SubSystem1208/4(Block3280)
  Block3274/1 -> SubSystem1261/4(Block3402)

== system lib_all/SubSystem1196/SubSystem1273 ==
  note: Text260
  note: Text259
  note: Text258
  Block3446 : Inport
  Block3447 : Inport  Port=2
  Block3448 : Inport  Port=3
  Block3449 : Inport  Port=4
  Block3450 : Inport  Port=5
  Block3451 : Product  OutDataTypeStr=double  SaturateOnIntegerOverflow=on  RndMeth=Zero  InputSameDT=on
  SubSystem1275 : Subsystem  Priority=1  TreatAsAtomicUnit=on
      settings: AttributesFormatString=Attribute681  RTWFcnNameOpts=Use subsystem name
  SubSystem1328 : Subsystem  Priority=2  TreatAsAtomicUnit=on
      settings: AttributesFormatString=Attribute700  RTWFcnNameOpts=Use subsystem name
  Block3620 : Outport
  wires:
  Block3446/1 -> SubSystem1328/1(Block3574), SubSystem1275/1(Block3452)
  Block3447/1 -> SubSystem1328/2(Block3575), SubSystem1275/2(Block3453)
  SubSystem1275/1(Block3573) -> Block3451/1  "SigLabel119"
  SubSystem1328/1(Block3619) -> Block3451/2  "SigLabel118"
  Block3451/1 -> Block3620/1
  Block3448/1 -> SubSystem1328/3(Block3576), SubSystem1275/3(Block3454)
  Block3450/1 -> SubSystem1275/4(Block3455)
  Block3449/1 -> SubSystem1328/4(Block3577)

== system lib_all/SubSystem1196/SubSystem1340 ==
  note: Text263
  Block3621 : Inport
  Block3622 : Inport  Port=2
  Block3623 : Inport  Port=3
  Block3624 : Inport  Port=4
  SubSystem1342 : Subsystem
  SubSystem1350 : Subsystem
  Block3666 : Outport
  wires:
  Block3622/1 -> SubSystem1342/1(Block3625)
  Block3621/1 -> SubSystem1342/2(Block3626)
  SubSystem1342/1(Block3657) -> SubSystem1350/2(Block3659)
  Block3624/1 -> SubSystem1350/1(Block3658)
  SubSystem1350/1(Block3665) -> Block3666/1
  Block3623/1 -> SubSystem1342/3(Block3627)

== system lib_all/SubSystem1196/SubSystem1352 ==
  note: Text266
  Block3667 : Inport
  Block3668 : Inport  Port=2
  Block3669 : Inport  Port=3
  Block3670 : Inport  Port=4
  SubSystem1354 : Subsystem
  SubSystem1362 : Subsystem
  Block3712 : Outport
  wires:
  Block3668/1 -> SubSystem1354/1(Block3671)
  Block3667/1 -> SubSystem1354/2(Block3672)
  SubSystem1354/1(Block3703) -> SubSystem1362/2(Block3705)
  Block3670/1 -> SubSystem1362/1(Block3704)
  SubSystem1362/1(Block3711) -> Block3712/1
  Block3669/1 -> SubSystem1354/3(Block3673)

== system lib_all/SubSystem1364/SubSystem1366 ==
  note: Text274
  Block3731 : Inport
  Block3732 : Inport  Port=2
  Block3733 : Inport  Port=3
  Block3734 : Inport  Port=4
  Block3735 : Constant  Value=0  OutDataTypeStr=double  SampleTime=-1
  Block3736 : Constant  OutDataTypeStr=double  SampleTime=-1
  Block3737 : Constant  Value=0  OutDataTypeStr=double  SampleTime=-1
  Block3738 : Gain  Gain=1/5  SaturateOnIntegerOverflow=on  OutDataTypeStr=Inherit: Same as input  ParamDataTypeStr=Inherit: Same as input
      settings: AttributesFormatString=Attribute747
  Block3739 : Product  OutDataTypeStr=double  SaturateOnIntegerOverflow=on  RndMeth=Zero  InputSameDT=on
  Block3740 : Sum  Inputs=-+  OutDataTypeStr=double  RndMeth=Zero  SaturateOnIntegerOverflow=on  InputSameDT=on
  Block3741 : Sum  OutDataTypeStr=double  RndMeth=Zero  SaturateOnIntegerOverflow=on  InputSameDT=on
  Block3742 : Sum  OutDataTypeStr=double  RndMeth=Zero  SaturateOnIntegerOverflow=on  InputSameDT=on
  Block3743 : Switch  Threshold=0.5  RndMeth=Zero  SaturateOnIntegerOverflow=on  InputSameDT=on
  SubSystem1368 : Subsystem
  SubSystem1369 : Subsystem
  SubSystem1375 : Subsystem
  Block3852 : Outport
  wires:
  Block3732/1 -> SubSystem1369/1(Block3744)
  SubSystem1369/1(Block3769) -> SubSystem1375/1(Block3771)
  Block3734/1 -> SubSystem1375/2(Block3772)
  SubSystem1375/1(Block3850) -> Block3741/1, Block3740/1
  SubSystem1375/2(Block3851) -> Block3740/2
  Block3740/1 -> Block3739/1  "SigLabel136"
  SubSystem1369/2(Block3770) -> Block3739/2
  Block3741/1 -> Block3743/3, SubSystem1368/1(In1)
  Block3733/1 -> SubSystem1369/3(Block3746)
  Block3737/1 -> SubSystem1368/2(In2)
  SubSystem1368/1(Out1) -> Block3743/2
  Block3743/1 -> Block3852/1
  Block3735/1 -> Block3743/1
  Block3736/1 -> Block3742/2
  Block3739/1 -> Block3738/1
  Block3738/1 -> Block3741/2
  Block3731/1 -> Block3742/1
  Block3742/1 -> SubSystem1369/2(Block3745), SubSystem1375/3(Block3773)

== system lib_all/SubSystem1364/SubSystem1419 ==
  note: Text280
  Block3853 : Inport
  Block3854 : Inport  Port=2
  Block3855 : Inport  Port=3
  Block3856 : Inport  Port=4
  Block3857 : Constant  Value=0  OutDataTypeStr=double  SampleTime=-1
  Block3858 : Constant  OutDataTypeStr=double  SampleTime=-1
  Block3859 : Constant  Value=0  OutDataTypeStr=double  SampleTime=-1
  Block3860 : Gain  Gain=1/5  SaturateOnIntegerOverflow=on  OutDataTypeStr=Inherit: Same as input  ParamDataTypeStr=Inherit: Same as input
      settings: AttributesFormatString=Attribute766
  Block3861 : Product  OutDataTypeStr=double  SaturateOnIntegerOverflow=on  RndMeth=Zero  InputSameDT=on
  Block3862 : Sum  Inputs=-+  OutDataTypeStr=double  RndMeth=Zero  SaturateOnIntegerOverflow=on  InputSameDT=on
  Block3863 : Sum  OutDataTypeStr=double  RndMeth=Zero  SaturateOnIntegerOverflow=on  InputSameDT=on
  Block3864 : Sum  OutDataTypeStr=double  RndMeth=Zero  SaturateOnIntegerOverflow=on  InputSameDT=on
  Block3865 : Switch  Threshold=0.5  RndMeth=Zero  SaturateOnIntegerOverflow=on  InputSameDT=on
  SubSystem1421 : Subsystem
  SubSystem1422 : Subsystem
  SubSystem1428 : Subsystem
  Block3974 : Outport
  wires:
  Block3854/1 -> SubSystem1422/1(Block3866)
  SubSystem1422/1(Block3891) -> SubSystem1428/1(Block3893)
  Block3856/1 -> SubSystem1428/2(Block3894)
  SubSystem1428/1(Block3972) -> Block3863/1, Block3862/1
  SubSystem1428/2(Block3973) -> Block3862/2
  Block3862/1 -> Block3861/1  "SigLabel139"
  SubSystem1422/2(Block3892) -> Block3861/2
  Block3863/1 -> Block3865/3, SubSystem1421/1(In1)
  Block3855/1 -> SubSystem1422/3(Block3868)
  Block3859/1 -> SubSystem1421/2(In2)
  SubSystem1421/1(Out1) -> Block3865/2
  Block3865/1 -> Block3974/1
  Block3857/1 -> Block3865/1
  Block3858/1 -> Block3864/2
  Block3861/1 -> Block3860/1
  Block3860/1 -> Block3863/2
  Block3853/1 -> Block3864/1
  Block3864/1 -> SubSystem1422/2(Block3867), SubSystem1428/3(Block3895)

== system lib_all/SubSystem1364/SubSystem1472 ==
  note: Text281
  Block3975 : Inport
  Block3976 : Constant  Value=0  OutDataTypeStr=double  SampleTime=-1
  Block3977 : Constant  Value=3  OutDataTypeStr=double  SampleTime=-1
  Block3978 : Constant  Value=2  OutDataTypeStr=double  SampleTime=-1
  Block3979 : Constant  Value=2  OutDataTypeStr=double  SampleTime=-1
  Block3980 : Constant  Value=0  OutDataTypeStr=double  SampleTime=-1
  Block3981 : Constant  OutDataTypeStr=double  SampleTime=-1
  Block3982 : DataStoreRead  DataStoreName=DSM48
  Block3983 : DataStoreRead  DataStoreName=DSM38
  Block3984 : DataStoreRead  DataStoreName=DSM37
  Block3985 : Demux  Outputs=2
  Block3986 : Mux  Inputs=2
  Block3987 : Mux  Inputs=2
  Block3988 : Mux  Inputs=2
  Block3989 : Switch  Threshold=0.5  RndMeth=Zero  SaturateOnIntegerOverflow=on  InputSameDT=on
  Block3990 : Switch  Threshold=0.5  RndMeth=Zero  SaturateOnIntegerOverflow=on  InputSameDT=on
  Block3991 : Switch  Threshold=0.5  RndMeth=Zero  SaturateOnIntegerOverflow=on  InputSameDT=on
  Block3992 : Lookup  InputValues=map_mr2_01  Table=map_mr2_02  SaturateOnIntegerOverflow=on
      settings: AttributesFormatString=<%<OutputValues>>\nAttribute787
  Block3993 : Lookup  InputValues=map_mr2_03  Table=map_mr2_04  SaturateOnIntegerOverflow=on
      settings: AttributesFormatString=<%<OutputValues>>\nAttribute788
  SubSystem1473 : Subsystem
  SubSystem1474 : Subsystem
  SubSystem1475 : Subsystem
  SubSystem1476 : Subsystem  TreatAsAtomicUnit=on  mask(***ATC.DSRVEC***){bd_version=1.01.A, r0=DSM97, r1=-1, r2=, r3=, r4=, r5=, r6=, r7=, r8=, r9=, c0=, c1=, c2=, c3=, c4=, c5=, c6=, c7=, c8=, c9=}
      mask prompts: bd_version "Version"
      mask help: これはAutoCからのブロックです．編集禁止．
      settings: OpenFcn=warndlg('変更禁止!');
      mask Help: web(ui_blockhelpmng(gcb));
      mask Display: disp('ATC.DSRVEC')
  SubSystem1477 : Subsystem  TreatAsAtomicUnit=on  mask(***ATC.DSRVEC***){bd_version=1.01.A, r0=DSM9, r1=-1, r2=, r3=, r4=, r5=, r6=, r7=, r8=, r9=, c0=, c1=, c2=, c3=, c4=, c5=, c6=, c7=, c8=, c9=}
      mask prompts: bd_version "Version"
      mask help: これはAutoCからのブロックです．編集禁止．
      settings: OpenFcn=warndlg('変更禁止!');
      mask Help: web(ui_blockhelpmng(gcb));
      mask Display: disp('ATC.DSRVEC')
  Block3994 : Outport
  Block3995 : Outport  Port=2
  wires:
  Block3982/1 -> SubSystem1473/1(In1), SubSystem1475/1(In1)
  Block3976/1 -> SubSystem1473/2(In2)
  Block3975/1 -> SubSystem1476/1(element), SubSystem1477/1(element)
  SubSystem1477/1(Output) -> Block3986/1
  Block3977/1 -> Block3986/2
  Block3984/1 -> Block3987/1
  Block3978/1 -> Block3987/2
  Block3979/1 -> Block3988/2
  SubSystem1476/1(Output) -> Block3992/1, Block3993/1
  Block3980/1 -> SubSystem1474/2(In2)
  Block3983/1 -> SubSystem1474/1(In1)
  SubSystem1474/1(Out1) -> Block3989/2
  Block3993/1 -> Block3989/1
  Block3992/1 -> Block3989/3
  SubSystem1473/1(Out1) -> Block3990/2
  Block3989/1 -> Block3988/1
  Block3981/1 -> SubSystem1475/2(In2)
  SubSystem1475/1(Out1) -> Block3991/2
  Block3987/1 -> Block3991/1
  Block3986/1 -> Block3991/3
  Block3988/1 -> Block3990/1
  Block3990/1 -> Block3985/1
  Block3985/1 -> Block3994/1
  Block3985/2 -> Block3995/1
  Block3991/1 -> Block3990/3

== system lib_all/SubSystem1364/SubSystem1478 ==
  note: Text284
  note: Text283
  note: Text282
  Block3996 : Inport
  Block3997 : Inport  Port=2
  Block3998 : Inport  Port=3
  Block3999 : Inport  Port=4
  Block4000 : DataStoreWrite  DataStoreName=DSM90
  Block4001 : DataStoreWrite  DataStoreName=DSM91
  Block4006 : Product  OutDataTypeStr=double  SaturateOnIntegerOverflow=on  RndMeth=Zero  InputSameDT=on
  Block4007 : Product  Inputs=*/  OutDataTypeStr=double  SaturateOnIntegerOverflow=on  RndMeth=Zero  InputSameDT=on
  Block4008 : Lookup2D  RowIndex=map_lib_15  ColumnIndex=map_lib_14  Table=map_lib_16  SaturateOnIntegerOverflow=on  InputSameDT=on
      settings: AttributesFormatString=<%<OutputValues>>\nAttribute789
  Block4009 : Lookup2D  RowIndex=map_lib_15  ColumnIndex=map_lib_14  Table=map_lib_16  SaturateOnIntegerOverflow=on  InputSameDT=on
      settings: AttributesFormatString=<%<OutputValues>>\nAttribute790
  Block4010 : Outport
  wires:
  Block3999/1 -> Block4008/2, Block4009/2
  [TagName20] -> Block4001/1
  [TagName19] -> Block4000/1
  Block4008/1 -> Block4007/2, [TagName19]
  Block4007/1 -> Block4010/1
  Block4006/1 -> Block4007/1
  Block3998/1 -> Block4006/2
  Block4009/1 -> Block4006/1, [TagName20]
  Block3996/1 -> Block4008/1
  Block3997/1 -> Block4009/1

== system lib_all/SubSystem1364/SubSystem1480 ==
  note: ***編集禁止***
  input : Inport
  element : Inport  Port=2
  Assignment : Assignment  IndexOptions=Index vector (port)  Indices=1  OutputSizes=[1 1]
  Inside_DSR : DataStoreRead  DataStoreName=DSM99
  Inside_DSW : DataStoreWrite  DataStoreName=DSM99
  wires:
  element/1 -> Assignment/3
  input/1 -> Assignment/2
  Inside_DSR/1 -> Assignment/1
  Assignment/1 -> Inside_DSW/1

== system lib_all/SubSystem1364/SubSystem1481 ==
  note: ***編集禁止***
  input : Inport
  element : Inport  Port=2
  Assignment : Assignment  IndexOptions=Index vector (port)  Indices=1  OutputSizes=[1 1]
  Inside_DSR : DataStoreRead  DataStoreName=DSM100
  Inside_DSW : DataStoreWrite  DataStoreName=DSM100
  wires:
  element/1 -> Assignment/3
  input/1 -> Assignment/2
  Inside_DSR/1 -> Assignment/1
  Assignment/1 -> Inside_DSW/1

== system lib_all/SubSystem1364/SubSystem1482 ==
  note: ***編集禁止***
  element : Inport
  Inside_DSR : DataStoreRead  DataStoreName=DSM98
  Selector : Selector  IndexOptions=Index vector (port)  Indices=[1 3]  OutputSizes=1
  Output : Outport
  wires:
  Selector/1 -> Output/1
  element/1 -> Selector/2
  Inside_DSR/1 -> Selector/1

== system lib_all/SubSystem1364/SubSystem1483 ==
  note: ***編集禁止***
  input : Inport
  element : Inport  Port=2
  Assignment : Assignment  IndexOptions=Index vector (port)  Indices=1  OutputSizes=[1 1]
  Inside_DSR : DataStoreRead  DataStoreName=DSM41
  Inside_DSW : DataStoreWrite  DataStoreName=DSM41
  wires:
  element/1 -> Assignment/3
  input/1 -> Assignment/2
  Inside_DSR/1 -> Assignment/1
  Assignment/1 -> Inside_DSW/1

== system lib_all/SubSystem1364/SubSystem1484 ==
  note: ***編集禁止***
  element : Inport
  Inside_DSR : DataStoreRead  DataStoreName=DSM42
  Selector : Selector  IndexOptions=Index vector (port)  Indices=[1 3]  OutputSizes=1
  Output : Outport
  wires:
  Selector/1 -> Output/1
  element/1 -> Selector/2
  Inside_DSR/1 -> Selector/1

== system lib_all/SubSystem1364/SubSystem1485 ==
  note: ***編集禁止***
  input : Inport
  element : Inport  Port=2
  Assignment : Assignment  IndexOptions=Index vector (port)  Indices=1  OutputSizes=[1 1]
  Inside_DSR : DataStoreRead  DataStoreName=DSM42
  Inside_DSW : DataStoreWrite  DataStoreName=DSM42
  wires:
  element/1 -> Assignment/3
  input/1 -> Assignment/2
  Inside_DSR/1 -> Assignment/1
  Assignment/1 -> Inside_DSW/1

== system lib_all/SubSystem1364/SubSystem1486 ==
  note: Text285
  Block4011 : Inport
  Block4012 : Inport  Port=2
  Block4013 : Inport  Port=3
  Block4014 : Constant  OutDataTypeStr=double  SampleTime=-1
  Block4015 : MinMax  Function=max  Inputs=2  RndMeth=Zero  SaturateOnIntegerOverflow=on  InputSameDT=on
  Block4016 : Product  Inputs=*/  OutDataTypeStr=double  SaturateOnIntegerOverflow=on  RndMeth=Zero  InputSameDT=on
      settings: AttributesFormatString=Attribute792
  Block4017 : Sum  Inputs=+-  OutDataTypeStr=double  RndMeth=Zero  SaturateOnIntegerOverflow=on  InputSameDT=on
  Block4018 : Sum  Inputs=2  OutDataTypeStr=double  RndMeth=Zero  SaturateOnIntegerOverflow=on  InputSameDT=on
  Block4019 : Outport
  wires:
  Block4015/1 -> Block4016/2
  Block4013/1 -> Block4015/1
  Block4014/1 -> Block4015/2
  Block4018/1 -> Block4019/1
  Block4016/1 -> Block4018/1
  Block4017/1 -> Block4016/1
  Block4012/1 -> Block4017/1
  Block4011/1 -> Block4017/2, Block4018/2

== system lib_all/SubSystem1487/SubSystem1489 ==
  note: ***編集禁止***
  In1 : Inport
  In2 : Inport  Port=2
  LO1 : Logic
  RO1 : RelationalOperator  Operator=<  InputSameDT=on
  RO2 : RelationalOperator  Operator=~=  InputSameDT=on
  single1 : DataTypeConversion  OutDataTypeStr=single  SaturateOnIntegerOverflow=on  RndMeth=Zero
  single2 : DataTypeConversion  OutDataTypeStr=single  SaturateOnIntegerOverflow=on  RndMeth=Zero
  Out1 : Outport
  wires:
  In2/1 -> single2/1
  In1/1 -> single1/1
  single1/1 -> RO1/1, RO2/1
  single2/1 -> RO1/2, RO2/2
  RO1/1 -> LO1/1
  RO2/1 -> LO1/2
  LO1/1 -> Out1/1
